simulink model "wlan_phy_rx_pmd"  (Simulink 7.8)
settings: Solver=VariableStepDiscrete  SolverMode=SingleTasking  StartTime=0.0  StopTime=rx_sim.sim_time  RelTol=1e-3
PostLoadFcn:
  | wlan_phy_rx_init
InitFcn:
  | wlan_phy_rx_init
StartFcn:
  | wlan_phy_rx_init
values from neighbouring files (symbols this model uses):
  barker_seq20 = 0.95 * circshift(barker_seq20, [0 4]) ./ max(abs(barker_seq20))    [wlan_phy_rx_init.m]
  bit_scrambler_lfsr_bytes = sum(bit_scrambler_lfsr_bytes .* repmat(2.^[0:7], 127, 1),2)    [wlan_phy_rx_init.m]
  longCorr_coef_i = [3*real(longCorr_coef)]    [wlan_phy_rx_init.m]
  longCorr_coef_q = [3*imag(longCorr_coef)]    [wlan_phy_rx_init.m]
  MAX_CP_LEN = 32    [wlan_phy_rx_init.m]
  MAX_NCBPS = NCBPS_11n_64QAM    (= 312)    [wlan_phy_rx_init.m]
  MAX_NUM_BYTES = 2^16-1    (= 65535)    [wlan_phy_rx_init.m]
  MAX_NUM_SC = 64    [wlan_phy_rx_init.m]
  MAX_NUM_SYMS = 600    [wlan_phy_rx_init.m]
  NDBPS_11a_BPSK12 = 24    [wlan_phy_rx_init.m]
  preFFT_sampBuff_numSamps = 4*MAX_NUM_SC    (= 256)    [wlan_phy_rx_init.m]
  rx_sim = struct()    [wlan_phy_rx_init.m]
  scr_ind_rev = zeros(1,128)    [wlan_phy_rx_init.m]
undefined symbols (not defined in the file or in its folder): gcbh, mcs_rom_11ag, mcs_rom_11n, wlan_rx_deinterleave_rom_gen, xlBlockLoadCallback, xlGetNormalizedPeriod, xlipgetpartsetting
libraries: xbsIndex_r4 x4943, xbsEDKLib_r4 x1
(part 25 of 28)

== system wlan_phy_rx_pmd/Sync & Antenna Sel/LTS Correlation/Correlator/C3/C3/MAC ==
  a : Inport
  coeff : Inport  Port=2
  ld : Inport  Port=3
  rst : Inport  Port=4
  AddSub : xbsIndex_r4/AddSub  mode=Addition or Subtraction  use_carryin=off  use_carryout=off  en=off  latency=0  precision=User Defined  arith_type="Signed  (2's comp)"  n_bits=long_cor_acc_n_bits  bin_pt=0  quantization=Truncate  overflow=Flag as error  dbl_ovrd=off  use_behavioral_HDL=on  hw_selection=Fabric  pipelined=off  use_rpm=off  block_type=addsub
      settings: xl_area=[2 0 0 4 0 0 0]  block_version=VER_STRING_GOES_HERE
  Gateway Out{1..3,6..8} (6 alike) : xbsIndex_r4/Gateway Out  inherit_from_input=off  hdl_port=off  timing_constraint=None  locs_specified=off  LOCs={}  UseAsDAC=off  DACChannel='1'  block_type=gatewayout
      settings: infoedit="Gateway out block.  Converts Xilinx fixed point inputs into ouputs of type Simulink integer, double, or fixed point.<P><P>Hardware notes:  In hardware these blocks become top level output ports or are discarded, depending on how they are configured."  xl_area=[0 0 0 0 0 0 0]  block_version=VER_STRING_GOES_HERE
  Register1 : xbsIndex_r4/Register  init=0  rst=on  en=off  dbl_ovrd=off  block_type=register
      settings: xl_area=[2 4 0 0 0 0 0]  block_version=VER_STRING_GOES_HERE
  Register2 : xbsIndex_r4/Register  init=0  rst=off  en=on  dbl_ovrd=off  block_type=register
      settings: xl_area=[2 4 0 0 0 0 0]  block_version=VER_STRING_GOES_HERE
  bc2 : xbsIndex_r4/Convert  gui_display_data_type=Fixed-point  arith_type=Boolean  n_bits=1  bin_pt=0  float_type=Single  exp_bits=8  fraction_bits=24  quantization=Truncate  overflow=Wrap  en=off  latency=0  dbl_ovrd=off  pipeline=off  block_type=convert
      settings: xl_area=[0 0 0 0 0 0 0]  block_version=VER_STRING_GOES_HERE
  bc3 : xbsIndex_r4/Convert  gui_display_data_type=Fixed-point  arith_type=Boolean  n_bits=1  bin_pt=0  float_type=Single  exp_bits=8  fraction_bits=24  quantization=Truncate  overflow=Wrap  en=off  latency=0  dbl_ovrd=off  pipeline=off  block_type=convert
      settings: xl_area=[0 0 0 0 0 0 0]  block_version=VER_STRING_GOES_HERE
  yn : Outport
  accrm : Outport  Port=2
  wires:
  rst/1 -> Gateway Out1/1, Register1/2
  Gateway Out3/1 -> ?
  Gateway Out2/1 -> ?
  Gateway Out1/1 -> ?
  Gateway Out6/1 -> ?
  Gateway Out7/1 -> ?
  Gateway Out8/1 -> ?
  a/1 -> Gateway Out7/1, bc2/1
  AddSub/1 -> Gateway Out8/1, accrm/1, Register1/1, Register2/1
  Register2/1 -> Gateway Out3/1, yn/1
  Register1/1 -> AddSub/1
  ld/1 -> Gateway Out2/1, bc3/1
  bc3/1 -> Register2/2
  bc2/1 -> AddSub/3
  coeff/1 -> Gateway Out6/1, AddSub/2

== system wlan_phy_rx_pmd/Sync & Antenna Sel/LTS Correlation/Correlator/C3/C4/MAC ==
  a : Inport
  coeff : Inport  Port=2
  ld : Inport  Port=3
  rst : Inport  Port=4
  AddSub : xbsIndex_r4/AddSub  mode=Addition or Subtraction  use_carryin=off  use_carryout=off  en=off  latency=0  precision=User Defined  arith_type="Signed  (2's comp)"  n_bits=long_cor_acc_n_bits  bin_pt=0  quantization=Truncate  overflow=Flag as error  dbl_ovrd=off  use_behavioral_HDL=on  hw_selection=Fabric  pipelined=off  use_rpm=off  block_type=addsub
      settings: xl_area=[2 0 0 4 0 0 0]  block_version=VER_STRING_GOES_HERE
  Gateway Out{1..3,6..8} (6 alike) : xbsIndex_r4/Gateway Out  inherit_from_input=off  hdl_port=off  timing_constraint=None  locs_specified=off  LOCs={}  UseAsDAC=off  DACChannel='1'  block_type=gatewayout
      settings: infoedit="Gateway out block.  Converts Xilinx fixed point inputs into ouputs of type Simulink integer, double, or fixed point.<P><P>Hardware notes:  In hardware these blocks become top level output ports or are discarded, depending on how they are configured."  xl_area=[0 0 0 0 0 0 0]  block_version=VER_STRING_GOES_HERE
  Register1 : xbsIndex_r4/Register  init=0  rst=on  en=off  dbl_ovrd=off  block_type=register
      settings: xl_area=[2 4 0 0 0 0 0]  block_version=VER_STRING_GOES_HERE
  Register2 : xbsIndex_r4/Register  init=0  rst=off  en=on  dbl_ovrd=off  block_type=register
      settings: xl_area=[2 4 0 0 0 0 0]  block_version=VER_STRING_GOES_HERE
  bc2 : xbsIndex_r4/Convert  gui_display_data_type=Fixed-point  arith_type=Boolean  n_bits=1  bin_pt=0  float_type=Single  exp_bits=8  fraction_bits=24  quantization=Truncate  overflow=Wrap  en=off  latency=0  dbl_ovrd=off  pipeline=off  block_type=convert
      settings: xl_area=[0 0 0 0 0 0 0]  block_version=VER_STRING_GOES_HERE
  bc3 : xbsIndex_r4/Convert  gui_display_data_type=Fixed-point  arith_type=Boolean  n_bits=1  bin_pt=0  float_type=Single  exp_bits=8  fraction_bits=24  quantization=Truncate  overflow=Wrap  en=off  latency=0  dbl_ovrd=off  pipeline=off  block_type=convert
      settings: xl_area=[0 0 0 0 0 0 0]  block_version=VER_STRING_GOES_HERE
  yn : Outport
  accrm : Outport  Port=2
  wires:
  coeff/1 -> AddSub/2, Gateway Out6/1
  bc2/1 -> AddSub/3
  bc3/1 -> Register2/2
  ld/1 -> bc3/1, Gateway Out2/1
  Register1/1 -> AddSub/1
  Register2/1 -> yn/1, Gateway Out3/1
  AddSub/1 -> Register2/1, Register1/1, accrm/1, Gateway Out8/1
  a/1 -> bc2/1, Gateway Out7/1
  Gateway Out8/1 -> ?
  Gateway Out7/1 -> ?
  Gateway Out6/1 -> ?
  Gateway Out1/1 -> ?
  Gateway Out2/1 -> ?
  Gateway Out3/1 -> ?
  rst/1 -> Register1/2, Gateway Out1/1

== system wlan_phy_rx_pmd/Sync & Antenna Sel/LTS Correlation/Correlator/C3/C5/MAC ==
  a : Inport
  coeff : Inport  Port=2
  ld : Inport  Port=3
  rst : Inport  Port=4
  AddSub : xbsIndex_r4/AddSub  mode=Addition or Subtraction  use_carryin=off  use_carryout=off  en=off  latency=0  precision=User Defined  arith_type="Signed  (2's comp)"  n_bits=long_cor_acc_n_bits  bin_pt=0  quantization=Truncate  overflow=Flag as error  dbl_ovrd=off  use_behavioral_HDL=on  hw_selection=Fabric  pipelined=off  use_rpm=off  block_type=addsub
      settings: xl_area=[2 0 0 4 0 0 0]  block_version=VER_STRING_GOES_HERE
  Gateway Out{1..3,6..8} (6 alike) : xbsIndex_r4/Gateway Out  inherit_from_input=off  hdl_port=off  timing_constraint=None  locs_specified=off  LOCs={}  UseAsDAC=off  DACChannel='1'  block_type=gatewayout
      settings: infoedit="Gateway out block.  Converts Xilinx fixed point inputs into ouputs of type Simulink integer, double, or fixed point.<P><P>Hardware notes:  In hardware these blocks become top level output ports or are discarded, depending on how they are configured."  xl_area=[0 0 0 0 0 0 0]  block_version=VER_STRING_GOES_HERE
  Register1 : xbsIndex_r4/Register  init=0  rst=on  en=off  dbl_ovrd=off  block_type=register
      settings: xl_area=[2 4 0 0 0 0 0]  block_version=VER_STRING_GOES_HERE
  Register2 : xbsIndex_r4/Register  init=0  rst=off  en=on  dbl_ovrd=off  block_type=register
      settings: xl_area=[2 4 0 0 0 0 0]  block_version=VER_STRING_GOES_HERE
  bc2 : xbsIndex_r4/Convert  gui_display_data_type=Fixed-point  arith_type=Boolean  n_bits=1  bin_pt=0  float_type=Single  exp_bits=8  fraction_bits=24  quantization=Truncate  overflow=Wrap  en=off  latency=0  dbl_ovrd=off  pipeline=off  block_type=convert
      settings: xl_area=[0 0 0 0 0 0 0]  block_version=VER_STRING_GOES_HERE
  bc3 : xbsIndex_r4/Convert  gui_display_data_type=Fixed-point  arith_type=Boolean  n_bits=1  bin_pt=0  float_type=Single  exp_bits=8  fraction_bits=24  quantization=Truncate  overflow=Wrap  en=off  latency=0  dbl_ovrd=off  pipeline=off  block_type=convert
      settings: xl_area=[0 0 0 0 0 0 0]  block_version=VER_STRING_GOES_HERE
  yn : Outport
  accrm : Outport  Port=2
  wires:
  rst/1 -> Gateway Out1/1, Register1/2
  Gateway Out3/1 -> ?
  Gateway Out2/1 -> ?
  Gateway Out1/1 -> ?
  Gateway Out6/1 -> ?
  Gateway Out7/1 -> ?
  Gateway Out8/1 -> ?
  a/1 -> Gateway Out7/1, bc2/1
  AddSub/1 -> Gateway Out8/1, accrm/1, Register1/1, Register2/1
  Register2/1 -> Gateway Out3/1, yn/1
  Register1/1 -> AddSub/1
  ld/1 -> Gateway Out2/1, bc3/1
  bc3/1 -> Register2/2
  bc2/1 -> AddSub/3
  coeff/1 -> Gateway Out6/1, AddSub/2

== system wlan_phy_rx_pmd/Sync & Antenna Sel/LTS Correlation/Correlator/C3/C6/MAC ==
  a : Inport
  coeff : Inport  Port=2
  ld : Inport  Port=3
  rst : Inport  Port=4
  AddSub : xbsIndex_r4/AddSub  mode=Addition or Subtraction  use_carryin=off  use_carryout=off  en=off  latency=0  precision=User Defined  arith_type="Signed  (2's comp)"  n_bits=long_cor_acc_n_bits  bin_pt=0  quantization=Truncate  overflow=Flag as error  dbl_ovrd=off  use_behavioral_HDL=on  hw_selection=Fabric  pipelined=off  use_rpm=off  block_type=addsub
      settings: xl_area=[2 0 0 4 0 0 0]  block_version=VER_STRING_GOES_HERE
  Gateway Out{1..3,6..8} (6 alike) : xbsIndex_r4/Gateway Out  inherit_from_input=off  hdl_port=off  timing_constraint=None  locs_specified=off  LOCs={}  UseAsDAC=off  DACChannel='1'  block_type=gatewayout
      settings: infoedit="Gateway out block.  Converts Xilinx fixed point inputs into ouputs of type Simulink integer, double, or fixed point.<P><P>Hardware notes:  In hardware these blocks become top level output ports or are discarded, depending on how they are configured."  xl_area=[0 0 0 0 0 0 0]  block_version=VER_STRING_GOES_HERE
  Register1 : xbsIndex_r4/Register  init=0  rst=on  en=off  dbl_ovrd=off  block_type=register
      settings: xl_area=[2 4 0 0 0 0 0]  block_version=VER_STRING_GOES_HERE
  Register2 : xbsIndex_r4/Register  init=0  rst=off  en=on  dbl_ovrd=off  block_type=register
      settings: xl_area=[2 4 0 0 0 0 0]  block_version=VER_STRING_GOES_HERE
  bc2 : xbsIndex_r4/Convert  gui_display_data_type=Fixed-point  arith_type=Boolean  n_bits=1  bin_pt=0  float_type=Single  exp_bits=8  fraction_bits=24  quantization=Truncate  overflow=Wrap  en=off  latency=0  dbl_ovrd=off  pipeline=off  block_type=convert
      settings: xl_area=[0 0 0 0 0 0 0]  block_version=VER_STRING_GOES_HERE
  bc3 : xbsIndex_r4/Convert  gui_display_data_type=Fixed-point  arith_type=Boolean  n_bits=1  bin_pt=0  float_type=Single  exp_bits=8  fraction_bits=24  quantization=Truncate  overflow=Wrap  en=off  latency=0  dbl_ovrd=off  pipeline=off  block_type=convert
      settings: xl_area=[0 0 0 0 0 0 0]  block_version=VER_STRING_GOES_HERE
  yn : Outport
  accrm : Outport  Port=2
  wires:
  coeff/1 -> AddSub/2, Gateway Out6/1
  bc2/1 -> AddSub/3
  bc3/1 -> Register2/2
  ld/1 -> bc3/1, Gateway Out2/1
  Register1/1 -> AddSub/1
  Register2/1 -> yn/1, Gateway Out3/1
  AddSub/1 -> Register2/1, Register1/1, accrm/1, Gateway Out8/1
  a/1 -> bc2/1, Gateway Out7/1
  Gateway Out8/1 -> ?
  Gateway Out7/1 -> ?
  Gateway Out6/1 -> ?
  Gateway Out1/1 -> ?
  Gateway Out2/1 -> ?
  Gateway Out3/1 -> ?
  rst/1 -> Register1/2, Gateway Out1/1

== system wlan_phy_rx_pmd/Sync & Antenna Sel/LTS Correlation/Correlator/C3/C7/MAC ==
  a : Inport
  coeff : Inport  Port=2
  ld : Inport  Port=3
  rst : Inport  Port=4
  AddSub : xbsIndex_r4/AddSub  mode=Addition or Subtraction  use_carryin=off  use_carryout=off  en=off  latency=0  precision=User Defined  arith_type="Signed  (2's comp)"  n_bits=long_cor_acc_n_bits  bin_pt=0  quantization=Truncate  overflow=Flag as error  dbl_ovrd=off  use_behavioral_HDL=on  hw_selection=Fabric  pipelined=off  use_rpm=off  block_type=addsub
      settings: xl_area=[2 0 0 4 0 0 0]  block_version=VER_STRING_GOES_HERE
  Gateway Out{1..3,6..8} (6 alike) : xbsIndex_r4/Gateway Out  inherit_from_input=off  hdl_port=off  timing_constraint=None  locs_specified=off  LOCs={}  UseAsDAC=off  DACChannel='1'  block_type=gatewayout
      settings: infoedit="Gateway out block.  Converts Xilinx fixed point inputs into ouputs of type Simulink integer, double, or fixed point.<P><P>Hardware notes:  In hardware these blocks become top level output ports or are discarded, depending on how they are configured."  xl_area=[0 0 0 0 0 0 0]  block_version=VER_STRING_GOES_HERE
  Register1 : xbsIndex_r4/Register  init=0  rst=on  en=off  dbl_ovrd=off  block_type=register
      settings: xl_area=[2 4 0 0 0 0 0]  block_version=VER_STRING_GOES_HERE
  Register2 : xbsIndex_r4/Register  init=0  rst=off  en=on  dbl_ovrd=off  block_type=register
      settings: xl_area=[2 4 0 0 0 0 0]  block_version=VER_STRING_GOES_HERE
  bc2 : xbsIndex_r4/Convert  gui_display_data_type=Fixed-point  arith_type=Boolean  n_bits=1  bin_pt=0  float_type=Single  exp_bits=8  fraction_bits=24  quantization=Truncate  overflow=Wrap  en=off  latency=0  dbl_ovrd=off  pipeline=off  block_type=convert
      settings: xl_area=[0 0 0 0 0 0 0]  block_version=VER_STRING_GOES_HERE
  bc3 : xbsIndex_r4/Convert  gui_display_data_type=Fixed-point  arith_type=Boolean  n_bits=1  bin_pt=0  float_type=Single  exp_bits=8  fraction_bits=24  quantization=Truncate  overflow=Wrap  en=off  latency=0  dbl_ovrd=off  pipeline=off  block_type=convert
      settings: xl_area=[0 0 0 0 0 0 0]  block_version=VER_STRING_GOES_HERE
  yn : Outport
  accrm : Outport  Port=2
  wires:
  rst/1 -> Gateway Out1/1, Register1/2
  Gateway Out3/1 -> ?
  Gateway Out2/1 -> ?
  Gateway Out1/1 -> ?
  Gateway Out6/1 -> ?
  Gateway Out7/1 -> ?
  Gateway Out8/1 -> ?
  a/1 -> Gateway Out7/1, bc2/1
  AddSub/1 -> Gateway Out8/1, accrm/1, Register1/1, Register2/1
  Register2/1 -> Gateway Out3/1, yn/1
  Register1/1 -> AddSub/1
  ld/1 -> Gateway Out2/1, bc3/1
  bc3/1 -> Register2/2
  bc2/1 -> AddSub/3
  coeff/1 -> Gateway Out6/1, AddSub/2

== system wlan_phy_rx_pmd/Sync & Antenna Sel/LTS Correlation/Correlator/C3/C8/MAC ==
  a : Inport
  coeff : Inport  Port=2
  ld : Inport  Port=3
  rst : Inport  Port=4
  AddSub : xbsIndex_r4/AddSub  mode=Addition or Subtraction  use_carryin=off  use_carryout=off  en=off  latency=0  precision=User Defined  arith_type="Signed  (2's comp)"  n_bits=long_cor_acc_n_bits  bin_pt=0  quantization=Truncate  overflow=Flag as error  dbl_ovrd=off  use_behavioral_HDL=on  hw_selection=Fabric  pipelined=off  use_rpm=off  block_type=addsub
      settings: xl_area=[2 0 0 4 0 0 0]  block_version=VER_STRING_GOES_HERE
  Gateway Out{1..3,6..8} (6 alike) : xbsIndex_r4/Gateway Out  inherit_from_input=off  hdl_port=off  timing_constraint=None  locs_specified=off  LOCs={}  UseAsDAC=off  DACChannel='1'  block_type=gatewayout
      settings: infoedit="Gateway out block.  Converts Xilinx fixed point inputs into ouputs of type Simulink integer, double, or fixed point.<P><P>Hardware notes:  In hardware these blocks become top level output ports or are discarded, depending on how they are configured."  xl_area=[0 0 0 0 0 0 0]  block_version=VER_STRING_GOES_HERE
  Register1 : xbsIndex_r4/Register  init=0  rst=on  en=off  dbl_ovrd=off  block_type=register
      settings: xl_area=[2 4 0 0 0 0 0]  block_version=VER_STRING_GOES_HERE
  Register2 : xbsIndex_r4/Register  init=0  rst=off  en=on  dbl_ovrd=off  block_type=register
      settings: xl_area=[2 4 0 0 0 0 0]  block_version=VER_STRING_GOES_HERE
  bc2 : xbsIndex_r4/Convert  gui_display_data_type=Fixed-point  arith_type=Boolean  n_bits=1  bin_pt=0  float_type=Single  exp_bits=8  fraction_bits=24  quantization=Truncate  overflow=Wrap  en=off  latency=0  dbl_ovrd=off  pipeline=off  block_type=convert
      settings: xl_area=[0 0 0 0 0 0 0]  block_version=VER_STRING_GOES_HERE
  bc3 : xbsIndex_r4/Convert  gui_display_data_type=Fixed-point  arith_type=Boolean  n_bits=1  bin_pt=0  float_type=Single  exp_bits=8  fraction_bits=24  quantization=Truncate  overflow=Wrap  en=off  latency=0  dbl_ovrd=off  pipeline=off  block_type=convert
      settings: xl_area=[0 0 0 0 0 0 0]  block_version=VER_STRING_GOES_HERE
  yn : Outport
  accrm : Outport  Port=2
  wires:
  coeff/1 -> AddSub/2, Gateway Out6/1
  bc2/1 -> AddSub/3
  bc3/1 -> Register2/2
  ld/1 -> bc3/1, Gateway Out2/1
  Register1/1 -> AddSub/1
  Register2/1 -> yn/1, Gateway Out3/1
  AddSub/1 -> Register2/1, Register1/1, accrm/1, Gateway Out8/1
  a/1 -> bc2/1, Gateway Out7/1
  Gateway Out8/1 -> ?
  Gateway Out7/1 -> ?
  Gateway Out6/1 -> ?
  Gateway Out1/1 -> ?
  Gateway Out2/1 -> ?
  Gateway Out3/1 -> ?
  rst/1 -> Register1/2, Gateway Out1/1

== system wlan_phy_rx_pmd/Sync & Antenna Sel/LTS Correlation/Correlator/C4/C1/MAC ==
  a : Inport
  coeff : Inport  Port=2
  ld : Inport  Port=3
  rst : Inport  Port=4
  AddSub : xbsIndex_r4/AddSub  mode=Addition or Subtraction  use_carryin=off  use_carryout=off  en=off  latency=0  precision=User Defined  arith_type="Signed  (2's comp)"  n_bits=long_cor_acc_n_bits  bin_pt=0  quantization=Truncate  overflow=Flag as error  dbl_ovrd=off  use_behavioral_HDL=on  hw_selection=Fabric  pipelined=off  use_rpm=off  block_type=addsub
      settings: xl_area=[2 0 0 4 0 0 0]  block_version=VER_STRING_GOES_HERE
  Gateway Out{1..3,6..8} (6 alike) : xbsIndex_r4/Gateway Out  inherit_from_input=off  hdl_port=off  timing_constraint=None  locs_specified=off  LOCs={}  UseAsDAC=off  DACChannel='1'  block_type=gatewayout
      settings: infoedit="Gateway out block.  Converts Xilinx fixed point inputs into ouputs of type Simulink integer, double, or fixed point.<P><P>Hardware notes:  In hardware these blocks become top level output ports or are discarded, depending on how they are configured."  xl_area=[0 0 0 0 0 0 0]  block_version=VER_STRING_GOES_HERE
  Register1 : xbsIndex_r4/Register  init=0  rst=on  en=off  dbl_ovrd=off  block_type=register
      settings: xl_area=[2 4 0 0 0 0 0]  block_version=VER_STRING_GOES_HERE
  Register2 : xbsIndex_r4/Register  init=0  rst=off  en=on  dbl_ovrd=off  block_type=register
      settings: xl_area=[2 4 0 0 0 0 0]  block_version=VER_STRING_GOES_HERE
  bc2 : xbsIndex_r4/Convert  gui_display_data_type=Fixed-point  arith_type=Boolean  n_bits=1  bin_pt=0  float_type=Single  exp_bits=8  fraction_bits=24  quantization=Truncate  overflow=Wrap  en=off  latency=0  dbl_ovrd=off  pipeline=off  block_type=convert
      settings: xl_area=[0 0 0 0 0 0 0]  block_version=VER_STRING_GOES_HERE
  bc3 : xbsIndex_r4/Convert  gui_display_data_type=Fixed-point  arith_type=Boolean  n_bits=1  bin_pt=0  float_type=Single  exp_bits=8  fraction_bits=24  quantization=Truncate  overflow=Wrap  en=off  latency=0  dbl_ovrd=off  pipeline=off  block_type=convert
      settings: xl_area=[0 0 0 0 0 0 0]  block_version=VER_STRING_GOES_HERE
  yn : Outport
  accrm : Outport  Port=2
  wires:
  coeff/1 -> AddSub/2, Gateway Out6/1
  bc2/1 -> AddSub/3
  bc3/1 -> Register2/2
  ld/1 -> bc3/1, Gateway Out2/1
  Register1/1 -> AddSub/1
  Register2/1 -> yn/1, Gateway Out3/1
  AddSub/1 -> Register2/1, Register1/1, accrm/1, Gateway Out8/1
  a/1 -> bc2/1, Gateway Out7/1
  Gateway Out8/1 -> ?
  Gateway Out7/1 -> ?
  Gateway Out6/1 -> ?
  Gateway Out1/1 -> ?
  Gateway Out2/1 -> ?
  Gateway Out3/1 -> ?
  rst/1 -> Register1/2, Gateway Out1/1

== system wlan_phy_rx_pmd/Sync & Antenna Sel/LTS Correlation/Correlator/C4/C2/MAC ==
  a : Inport
  coeff : Inport  Port=2
  ld : Inport  Port=3
  rst : Inport  Port=4
  AddSub : xbsIndex_r4/AddSub  mode=Addition or Subtraction  use_carryin=off  use_carryout=off  en=off  latency=0  precision=User Defined  arith_type="Signed  (2's comp)"  n_bits=long_cor_acc_n_bits  bin_pt=0  quantization=Truncate  overflow=Flag as error  dbl_ovrd=off  use_behavioral_HDL=on  hw_selection=Fabric  pipelined=off  use_rpm=off  block_type=addsub
      settings: xl_area=[2 0 0 4 0 0 0]  block_version=VER_STRING_GOES_HERE
  Gateway Out{1..3,6..8} (6 alike) : xbsIndex_r4/Gateway Out  inherit_from_input=off  hdl_port=off  timing_constraint=None  locs_specified=off  LOCs={}  UseAsDAC=off  DACChannel='1'  block_type=gatewayout
      settings: infoedit="Gateway out block.  Converts Xilinx fixed point inputs into ouputs of type Simulink integer, double, or fixed point.<P><P>Hardware notes:  In hardware these blocks become top level output ports or are discarded, depending on how they are configured."  xl_area=[0 0 0 0 0 0 0]  block_version=VER_STRING_GOES_HERE
  Register1 : xbsIndex_r4/Register  init=0  rst=on  en=off  dbl_ovrd=off  block_type=register
      settings: xl_area=[2 4 0 0 0 0 0]  block_version=VER_STRING_GOES_HERE
  Register2 : xbsIndex_r4/Register  init=0  rst=off  en=on  dbl_ovrd=off  block_type=register
      settings: xl_area=[2 4 0 0 0 0 0]  block_version=VER_STRING_GOES_HERE
  bc2 : xbsIndex_r4/Convert  gui_display_data_type=Fixed-point  arith_type=Boolean  n_bits=1  bin_pt=0  float_type=Single  exp_bits=8  fraction_bits=24  quantization=Truncate  overflow=Wrap  en=off  latency=0  dbl_ovrd=off  pipeline=off  block_type=convert
      settings: xl_area=[0 0 0 0 0 0 0]  block_version=VER_STRING_GOES_HERE
  bc3 : xbsIndex_r4/Convert  gui_display_data_type=Fixed-point  arith_type=Boolean  n_bits=1  bin_pt=0  float_type=Single  exp_bits=8  fraction_bits=24  quantization=Truncate  overflow=Wrap  en=off  latency=0  dbl_ovrd=off  pipeline=off  block_type=convert
      settings: xl_area=[0 0 0 0 0 0 0]  block_version=VER_STRING_GOES_HERE
  yn : Outport
  accrm : Outport  Port=2
  wires:
  rst/1 -> Gateway Out1/1, Register1/2
  Gateway Out3/1 -> ?
  Gateway Out2/1 -> ?
  Gateway Out1/1 -> ?
  Gateway Out6/1 -> ?
  Gateway Out7/1 -> ?
  Gateway Out8/1 -> ?
  a/1 -> Gateway Out7/1, bc2/1
  AddSub/1 -> Gateway Out8/1, accrm/1, Register1/1, Register2/1
  Register2/1 -> Gateway Out3/1, yn/1
  Register1/1 -> AddSub/1
  ld/1 -> Gateway Out2/1, bc3/1
  bc3/1 -> Register2/2
  bc2/1 -> AddSub/3
  coeff/1 -> Gateway Out6/1, AddSub/2

== system wlan_phy_rx_pmd/Sync & Antenna Sel/LTS Correlation/Correlator/C4/C3/MAC ==
  a : Inport
  coeff : Inport  Port=2
  ld : Inport  Port=3
  rst : Inport  Port=4
  AddSub : xbsIndex_r4/AddSub  mode=Addition or Subtraction  use_carryin=off  use_carryout=off  en=off  latency=0  precision=User Defined  arith_type="Signed  (2's comp)"  n_bits=long_cor_acc_n_bits  bin_pt=0  quantization=Truncate  overflow=Flag as error  dbl_ovrd=off  use_behavioral_HDL=on  hw_selection=Fabric  pipelined=off  use_rpm=off  block_type=addsub
      settings: xl_area=[2 0 0 4 0 0 0]  block_version=VER_STRING_GOES_HERE
  Gateway Out{1..3,6..8} (6 alike) : xbsIndex_r4/Gateway Out  inherit_from_input=off  hdl_port=off  timing_constraint=None  locs_specified=off  LOCs={}  UseAsDAC=off  DACChannel='1'  block_type=gatewayout
      settings: infoedit="Gateway out block.  Converts Xilinx fixed point inputs into ouputs of type Simulink integer, double, or fixed point.<P><P>Hardware notes:  In hardware these blocks become top level output ports or are discarded, depending on how they are configured."  xl_area=[0 0 0 0 0 0 0]  block_version=VER_STRING_GOES_HERE
  Register1 : xbsIndex_r4/Register  init=0  rst=on  en=off  dbl_ovrd=off  block_type=register
      settings: xl_area=[2 4 0 0 0 0 0]  block_version=VER_STRING_GOES_HERE
  Register2 : xbsIndex_r4/Register  init=0  rst=off  en=on  dbl_ovrd=off  block_type=register
      settings: xl_area=[2 4 0 0 0 0 0]  block_version=VER_STRING_GOES_HERE
  bc2 : xbsIndex_r4/Convert  gui_display_data_type=Fixed-point  arith_type=Boolean  n_bits=1  bin_pt=0  float_type=Single  exp_bits=8  fraction_bits=24  quantization=Truncate  overflow=Wrap  en=off  latency=0  dbl_ovrd=off  pipeline=off  block_type=convert
      settings: xl_area=[0 0 0 0 0 0 0]  block_version=VER_STRING_GOES_HERE
  bc3 : xbsIndex_r4/Convert  gui_display_data_type=Fixed-point  arith_type=Boolean  n_bits=1  bin_pt=0  float_type=Single  exp_bits=8  fraction_bits=24  quantization=Truncate  overflow=Wrap  en=off  latency=0  dbl_ovrd=off  pipeline=off  block_type=convert
      settings: xl_area=[0 0 0 0 0 0 0]  block_version=VER_STRING_GOES_HERE
  yn : Outport
  accrm : Outport  Port=2
  wires:
  coeff/1 -> AddSub/2, Gateway Out6/1
  bc2/1 -> AddSub/3
  bc3/1 -> Register2/2
  ld/1 -> bc3/1, Gateway Out2/1
  Register1/1 -> AddSub/1
  Register2/1 -> yn/1, Gateway Out3/1
  AddSub/1 -> Register2/1, Register1/1, accrm/1, Gateway Out8/1
  a/1 -> bc2/1, Gateway Out7/1
  Gateway Out8/1 -> ?
  Gateway Out7/1 -> ?
  Gateway Out6/1 -> ?
  Gateway Out1/1 -> ?
  Gateway Out2/1 -> ?
  Gateway Out3/1 -> ?
  rst/1 -> Register1/2, Gateway Out1/1

== system wlan_phy_rx_pmd/Sync & Antenna Sel/LTS Correlation/Correlator/C4/C4/MAC ==
  a : Inport
  coeff : Inport  Port=2
  ld : Inport  Port=3
  rst : Inport  Port=4
  AddSub : xbsIndex_r4/AddSub  mode=Addition or Subtraction  use_carryin=off  use_carryout=off  en=off  latency=0  precision=User Defined  arith_type="Signed  (2's comp)"  n_bits=long_cor_acc_n_bits  bin_pt=0  quantization=Truncate  overflow=Flag as error  dbl_ovrd=off  use_behavioral_HDL=on  hw_selection=Fabric  pipelined=off  use_rpm=off  block_type=addsub
      settings: xl_area=[2 0 0 4 0 0 0]  block_version=VER_STRING_GOES_HERE
  Gateway Out{1..3,6..8} (6 alike) : xbsIndex_r4/Gateway Out  inherit_from_input=off  hdl_port=off  timing_constraint=None  locs_specified=off  LOCs={}  UseAsDAC=off  DACChannel='1'  block_type=gatewayout
      settings: infoedit="Gateway out block.  Converts Xilinx fixed point inputs into ouputs of type Simulink integer, double, or fixed point.<P><P>Hardware notes:  In hardware these blocks become top level output ports or are discarded, depending on how they are configured."  xl_area=[0 0 0 0 0 0 0]  block_version=VER_STRING_GOES_HERE
  Register1 : xbsIndex_r4/Register  init=0  rst=on  en=off  dbl_ovrd=off  block_type=register
      settings: xl_area=[2 4 0 0 0 0 0]  block_version=VER_STRING_GOES_HERE
  Register2 : xbsIndex_r4/Register  init=0  rst=off  en=on  dbl_ovrd=off  block_type=register
      settings: xl_area=[2 4 0 0 0 0 0]  block_version=VER_STRING_GOES_HERE
  bc2 : xbsIndex_r4/Convert  gui_display_data_type=Fixed-point  arith_type=Boolean  n_bits=1  bin_pt=0  float_type=Single  exp_bits=8  fraction_bits=24  quantization=Truncate  overflow=Wrap  en=off  latency=0  dbl_ovrd=off  pipeline=off  block_type=convert
      settings: xl_area=[0 0 0 0 0 0 0]  block_version=VER_STRING_GOES_HERE
  bc3 : xbsIndex_r4/Convert  gui_display_data_type=Fixed-point  arith_type=Boolean  n_bits=1  bin_pt=0  float_type=Single  exp_bits=8  fraction_bits=24  quantization=Truncate  overflow=Wrap  en=off  latency=0  dbl_ovrd=off  pipeline=off  block_type=convert
      settings: xl_area=[0 0 0 0 0 0 0]  block_version=VER_STRING_GOES_HERE
  yn : Outport
  accrm : Outport  Port=2
  wires:
  rst/1 -> Gateway Out1/1, Register1/2
  Gateway Out3/1 -> ?
  Gateway Out2/1 -> ?
  Gateway Out1/1 -> ?
  Gateway Out6/1 -> ?
  Gateway Out7/1 -> ?
  Gateway Out8/1 -> ?
  a/1 -> Gateway Out7/1, bc2/1
  AddSub/1 -> Gateway Out8/1, accrm/1, Register1/1, Register2/1
  Register2/1 -> Gateway Out3/1, yn/1
  Register1/1 -> AddSub/1
  ld/1 -> Gateway Out2/1, bc3/1
  bc3/1 -> Register2/2
  bc2/1 -> AddSub/3
  coeff/1 -> Gateway Out6/1, AddSub/2

== system wlan_phy_rx_pmd/Sync & Antenna Sel/LTS Correlation/Correlator/C4/C5/MAC ==
  a : Inport
  coeff : Inport  Port=2
  ld : Inport  Port=3
  rst : Inport  Port=4
  AddSub : xbsIndex_r4/AddSub  mode=Addition or Subtraction  use_carryin=off  use_carryout=off  en=off  latency=0  precision=User Defined  arith_type="Signed  (2's comp)"  n_bits=long_cor_acc_n_bits  bin_pt=0  quantization=Truncate  overflow=Flag as error  dbl_ovrd=off  use_behavioral_HDL=on  hw_selection=Fabric  pipelined=off  use_rpm=off  block_type=addsub
      settings: xl_area=[2 0 0 4 0 0 0]  block_version=VER_STRING_GOES_HERE
  Gateway Out{1..3,6..8} (6 alike) : xbsIndex_r4/Gateway Out  inherit_from_input=off  hdl_port=off  timing_constraint=None  locs_specified=off  LOCs={}  UseAsDAC=off  DACChannel='1'  block_type=gatewayout
      settings: infoedit="Gateway out block.  Converts Xilinx fixed point inputs into ouputs of type Simulink integer, double, or fixed point.<P><P>Hardware notes:  In hardware these blocks become top level output ports or are discarded, depending on how they are configured."  xl_area=[0 0 0 0 0 0 0]  block_version=VER_STRING_GOES_HERE
  Register1 : xbsIndex_r4/Register  init=0  rst=on  en=off  dbl_ovrd=off  block_type=register
      settings: xl_area=[2 4 0 0 0 0 0]  block_version=VER_STRING_GOES_HERE
  Register2 : xbsIndex_r4/Register  init=0  rst=off  en=on  dbl_ovrd=off  block_type=register
      settings: xl_area=[2 4 0 0 0 0 0]  block_version=VER_STRING_GOES_HERE
  bc2 : xbsIndex_r4/Convert  gui_display_data_type=Fixed-point  arith_type=Boolean  n_bits=1  bin_pt=0  float_type=Single  exp_bits=8  fraction_bits=24  quantization=Truncate  overflow=Wrap  en=off  latency=0  dbl_ovrd=off  pipeline=off  block_type=convert
      settings: xl_area=[0 0 0 0 0 0 0]  block_version=VER_STRING_GOES_HERE
  bc3 : xbsIndex_r4/Convert  gui_display_data_type=Fixed-point  arith_type=Boolean  n_bits=1  bin_pt=0  float_type=Single  exp_bits=8  fraction_bits=24  quantization=Truncate  overflow=Wrap  en=off  latency=0  dbl_ovrd=off  pipeline=off  block_type=convert
      settings: xl_area=[0 0 0 0 0 0 0]  block_version=VER_STRING_GOES_HERE
  yn : Outport
  accrm : Outport  Port=2
  wires:
  coeff/1 -> AddSub/2, Gateway Out6/1
  bc2/1 -> AddSub/3
  bc3/1 -> Register2/2
  ld/1 -> bc3/1, Gateway Out2/1
  Register1/1 -> AddSub/1
  Register2/1 -> yn/1, Gateway Out3/1
  AddSub/1 -> Register2/1, Register1/1, accrm/1, Gateway Out8/1
  a/1 -> bc2/1, Gateway Out7/1
  Gateway Out8/1 -> ?
  Gateway Out7/1 -> ?
  Gateway Out6/1 -> ?
  Gateway Out1/1 -> ?
  Gateway Out2/1 -> ?
  Gateway Out3/1 -> ?
  rst/1 -> Register1/2, Gateway Out1/1

== system wlan_phy_rx_pmd/Sync & Antenna Sel/LTS Correlation/Correlator/C4/C6/MAC ==
  a : Inport
  coeff : Inport  Port=2
  ld : Inport  Port=3
  rst : Inport  Port=4
  AddSub : xbsIndex_r4/AddSub  mode=Addition or Subtraction  use_carryin=off  use_carryout=off  en=off  latency=0  precision=User Defined  arith_type="Signed  (2's comp)"  n_bits=long_cor_acc_n_bits  bin_pt=0  quantization=Truncate  overflow=Flag as error  dbl_ovrd=off  use_behavioral_HDL=on  hw_selection=Fabric  pipelined=off  use_rpm=off  block_type=addsub
      settings: xl_area=[2 0 0 4 0 0 0]  block_version=VER_STRING_GOES_HERE
  Gateway Out{1..3,6..8} (6 alike) : xbsIndex_r4/Gateway Out  inherit_from_input=off  hdl_port=off  timing_constraint=None  locs_specified=off  LOCs={}  UseAsDAC=off  DACChannel='1'  block_type=gatewayout
      settings: infoedit="Gateway out block.  Converts Xilinx fixed point inputs into ouputs of type Simulink integer, double, or fixed point.<P><P>Hardware notes:  In hardware these blocks become top level output ports or are discarded, depending on how they are configured."  xl_area=[0 0 0 0 0 0 0]  block_version=VER_STRING_GOES_HERE
  Register1 : xbsIndex_r4/Register  init=0  rst=on  en=off  dbl_ovrd=off  block_type=register
      settings: xl_area=[2 4 0 0 0 0 0]  block_version=VER_STRING_GOES_HERE
  Register2 : xbsIndex_r4/Register  init=0  rst=off  en=on  dbl_ovrd=off  block_type=register
      settings: xl_area=[2 4 0 0 0 0 0]  block_version=VER_STRING_GOES_HERE
  bc2 : xbsIndex_r4/Convert  gui_display_data_type=Fixed-point  arith_type=Boolean  n_bits=1  bin_pt=0  float_type=Single  exp_bits=8  fraction_bits=24  quantization=Truncate  overflow=Wrap  en=off  latency=0  dbl_ovrd=off  pipeline=off  block_type=convert
      settings: xl_area=[0 0 0 0 0 0 0]  block_version=VER_STRING_GOES_HERE
  bc3 : xbsIndex_r4/Convert  gui_display_data_type=Fixed-point  arith_type=Boolean  n_bits=1  bin_pt=0  float_type=Single  exp_bits=8  fraction_bits=24  quantization=Truncate  overflow=Wrap  en=off  latency=0  dbl_ovrd=off  pipeline=off  block_type=convert
      settings: xl_area=[0 0 0 0 0 0 0]  block_version=VER_STRING_GOES_HERE
  yn : Outport
  accrm : Outport  Port=2
  wires:
  rst/1 -> Gateway Out1/1, Register1/2
  Gateway Out3/1 -> ?
  Gateway Out2/1 -> ?
  Gateway Out1/1 -> ?
  Gateway Out6/1 -> ?
  Gateway Out7/1 -> ?
  Gateway Out8/1 -> ?
  a/1 -> Gateway Out7/1, bc2/1
  AddSub/1 -> Gateway Out8/1, accrm/1, Register1/1, Register2/1
  Register2/1 -> Gateway Out3/1, yn/1
  Register1/1 -> AddSub/1
  ld/1 -> Gateway Out2/1, bc3/1
  bc3/1 -> Register2/2
  bc2/1 -> AddSub/3
  coeff/1 -> Gateway Out6/1, AddSub/2

== system wlan_phy_rx_pmd/Sync & Antenna Sel/LTS Correlation/Correlator/C4/C7/MAC ==
  a : Inport
  coeff : Inport  Port=2
  ld : Inport  Port=3
  rst : Inport  Port=4
  AddSub : xbsIndex_r4/AddSub  mode=Addition or Subtraction  use_carryin=off  use_carryout=off  en=off  latency=0  precision=User Defined  arith_type="Signed  (2's comp)"  n_bits=long_cor_acc_n_bits  bin_pt=0  quantization=Truncate  overflow=Flag as error  dbl_ovrd=off  use_behavioral_HDL=on  hw_selection=Fabric  pipelined=off  use_rpm=off  block_type=addsub
      settings: xl_area=[2 0 0 4 0 0 0]  block_version=VER_STRING_GOES_HERE
  Gateway Out{1..3,6..8} (6 alike) : xbsIndex_r4/Gateway Out  inherit_from_input=off  hdl_port=off  timing_constraint=None  locs_specified=off  LOCs={}  UseAsDAC=off  DACChannel='1'  block_type=gatewayout
      settings: infoedit="Gateway out block.  Converts Xilinx fixed point inputs into ouputs of type Simulink integer, double, or fixed point.<P><P>Hardware notes:  In hardware these blocks become top level output ports or are discarded, depending on how they are configured."  xl_area=[0 0 0 0 0 0 0]  block_version=VER_STRING_GOES_HERE
  Register1 : xbsIndex_r4/Register  init=0  rst=on  en=off  dbl_ovrd=off  block_type=register
      settings: xl_area=[2 4 0 0 0 0 0]  block_version=VER_STRING_GOES_HERE
  Register2 : xbsIndex_r4/Register  init=0  rst=off  en=on  dbl_ovrd=off  block_type=register
      settings: xl_area=[2 4 0 0 0 0 0]  block_version=VER_STRING_GOES_HERE
  bc2 : xbsIndex_r4/Convert  gui_display_data_type=Fixed-point  arith_type=Boolean  n_bits=1  bin_pt=0  float_type=Single  exp_bits=8  fraction_bits=24  quantization=Truncate  overflow=Wrap  en=off  latency=0  dbl_ovrd=off  pipeline=off  block_type=convert
      settings: xl_area=[0 0 0 0 0 0 0]  block_version=VER_STRING_GOES_HERE
  bc3 : xbsIndex_r4/Convert  gui_display_data_type=Fixed-point  arith_type=Boolean  n_bits=1  bin_pt=0  float_type=Single  exp_bits=8  fraction_bits=24  quantization=Truncate  overflow=Wrap  en=off  latency=0  dbl_ovrd=off  pipeline=off  block_type=convert
      settings: xl_area=[0 0 0 0 0 0 0]  block_version=VER_STRING_GOES_HERE
  yn : Outport
  accrm : Outport  Port=2
  wires:
  coeff/1 -> AddSub/2, Gateway Out6/1
  bc2/1 -> AddSub/3
  bc3/1 -> Register2/2
  ld/1 -> bc3/1, Gateway Out2/1
  Register1/1 -> AddSub/1
  Register2/1 -> yn/1, Gateway Out3/1
  AddSub/1 -> Register2/1, Register1/1, accrm/1, Gateway Out8/1
  a/1 -> bc2/1, Gateway Out7/1
  Gateway Out8/1 -> ?
  Gateway Out7/1 -> ?
  Gateway Out6/1 -> ?
  Gateway Out1/1 -> ?
  Gateway Out2/1 -> ?
  Gateway Out3/1 -> ?
  rst/1 -> Register1/2, Gateway Out1/1

== system wlan_phy_rx_pmd/Sync & Antenna Sel/LTS Correlation/Correlator/C4/C8/MAC ==
  a : Inport
  coeff : Inport  Port=2
  ld : Inport  Port=3
  rst : Inport  Port=4
  AddSub : xbsIndex_r4/AddSub  mode=Addition or Subtraction  use_carryin=off  use_carryout=off  en=off  latency=0  precision=User Defined  arith_type="Signed  (2's comp)"  n_bits=long_cor_acc_n_bits  bin_pt=0  quantization=Truncate  overflow=Flag as error  dbl_ovrd=off  use_behavioral_HDL=on  hw_selection=Fabric  pipelined=off  use_rpm=off  block_type=addsub
      settings: xl_area=[2 0 0 4 0 0 0]  block_version=VER_STRING_GOES_HERE
  Gateway Out{1..3,6..8} (6 alike) : xbsIndex_r4/Gateway Out  inherit_from_input=off  hdl_port=off  timing_constraint=None  locs_specified=off  LOCs={}  UseAsDAC=off  DACChannel='1'  block_type=gatewayout
      settings: infoedit="Gateway out block.  Converts Xilinx fixed point inputs into ouputs of type Simulink integer, double, or fixed point.<P><P>Hardware notes:  In hardware these blocks become top level output ports or are discarded, depending on how they are configured."  xl_area=[0 0 0 0 0 0 0]  block_version=VER_STRING_GOES_HERE
  Register1 : xbsIndex_r4/Register  init=0  rst=on  en=off  dbl_ovrd=off  block_type=register
      settings: xl_area=[2 4 0 0 0 0 0]  block_version=VER_STRING_GOES_HERE
  Register2 : xbsIndex_r4/Register  init=0  rst=off  en=on  dbl_ovrd=off  block_type=register
      settings: xl_area=[2 4 0 0 0 0 0]  block_version=VER_STRING_GOES_HERE
  bc2 : xbsIndex_r4/Convert  gui_display_data_type=Fixed-point  arith_type=Boolean  n_bits=1  bin_pt=0  float_type=Single  exp_bits=8  fraction_bits=24  quantization=Truncate  overflow=Wrap  en=off  latency=0  dbl_ovrd=off  pipeline=off  block_type=convert
      settings: xl_area=[0 0 0 0 0 0 0]  block_version=VER_STRING_GOES_HERE
  bc3 : xbsIndex_r4/Convert  gui_display_data_type=Fixed-point  arith_type=Boolean  n_bits=1  bin_pt=0  float_type=Single  exp_bits=8  fraction_bits=24  quantization=Truncate  overflow=Wrap  en=off  latency=0  dbl_ovrd=off  pipeline=off  block_type=convert
      settings: xl_area=[0 0 0 0 0 0 0]  block_version=VER_STRING_GOES_HERE
  yn : Outport
  accrm : Outport  Port=2
  wires:
  rst/1 -> Gateway Out1/1, Register1/2
  Gateway Out3/1 -> ?
  Gateway Out2/1 -> ?
  Gateway Out1/1 -> ?
  Gateway Out6/1 -> ?
  Gateway Out7/1 -> ?
  Gateway Out8/1 -> ?
  a/1 -> Gateway Out7/1, bc2/1
  AddSub/1 -> Gateway Out8/1, accrm/1, Register1/1, Register2/1
  Register2/1 -> Gateway Out3/1, yn/1
  Register1/1 -> AddSub/1
  ld/1 -> Gateway Out2/1, bc3/1
  bc3/1 -> Register2/2
  bc2/1 -> AddSub/3
  coeff/1 -> Gateway Out6/1, AddSub/2

== system wlan_phy_rx_pmd/Sync & Antenna Sel/LTS Correlation/Correlator/C5/C1/MAC ==
  a : Inport
  coeff : Inport  Port=2
  ld : Inport  Port=3
  rst : Inport  Port=4
  AddSub : xbsIndex_r4/AddSub  mode=Addition or Subtraction  use_carryin=off  use_carryout=off  en=off  latency=0  precision=User Defined  arith_type="Signed  (2's comp)"  n_bits=long_cor_acc_n_bits  bin_pt=0  quantization=Truncate  overflow=Flag as error  dbl_ovrd=off  use_behavioral_HDL=on  hw_selection=Fabric  pipelined=off  use_rpm=off  block_type=addsub
      settings: xl_area=[2 0 0 4 0 0 0]  block_version=VER_STRING_GOES_HERE
  Gateway Out{1..3,6..8} (6 alike) : xbsIndex_r4/Gateway Out  inherit_from_input=off  hdl_port=off  timing_constraint=None  locs_specified=off  LOCs={}  UseAsDAC=off  DACChannel='1'  block_type=gatewayout
      settings: infoedit="Gateway out block.  Converts Xilinx fixed point inputs into ouputs of type Simulink integer, double, or fixed point.<P><P>Hardware notes:  In hardware these blocks become top level output ports or are discarded, depending on how they are configured."  xl_area=[0 0 0 0 0 0 0]  block_version=VER_STRING_GOES_HERE
  Register1 : xbsIndex_r4/Register  init=0  rst=on  en=off  dbl_ovrd=off  block_type=register
      settings: xl_area=[2 4 0 0 0 0 0]  block_version=VER_STRING_GOES_HERE
  Register2 : xbsIndex_r4/Register  init=0  rst=off  en=on  dbl_ovrd=off  block_type=register
      settings: xl_area=[2 4 0 0 0 0 0]  block_version=VER_STRING_GOES_HERE
  bc2 : xbsIndex_r4/Convert  gui_display_data_type=Fixed-point  arith_type=Boolean  n_bits=1  bin_pt=0  float_type=Single  exp_bits=8  fraction_bits=24  quantization=Truncate  overflow=Wrap  en=off  latency=0  dbl_ovrd=off  pipeline=off  block_type=convert
      settings: xl_area=[0 0 0 0 0 0 0]  block_version=VER_STRING_GOES_HERE
  bc3 : xbsIndex_r4/Convert  gui_display_data_type=Fixed-point  arith_type=Boolean  n_bits=1  bin_pt=0  float_type=Single  exp_bits=8  fraction_bits=24  quantization=Truncate  overflow=Wrap  en=off  latency=0  dbl_ovrd=off  pipeline=off  block_type=convert
      settings: xl_area=[0 0 0 0 0 0 0]  block_version=VER_STRING_GOES_HERE
  yn : Outport
  accrm : Outport  Port=2
  wires:
  rst/1 -> Gateway Out1/1, Register1/2
  Gateway Out3/1 -> ?
  Gateway Out2/1 -> ?
  Gateway Out1/1 -> ?
  Gateway Out6/1 -> ?
  Gateway Out7/1 -> ?
  Gateway Out8/1 -> ?
  a/1 -> Gateway Out7/1, bc2/1
  AddSub/1 -> Gateway Out8/1, accrm/1, Register1/1, Register2/1
  Register2/1 -> Gateway Out3/1, yn/1
  Register1/1 -> AddSub/1
  ld/1 -> Gateway Out2/1, bc3/1
  bc3/1 -> Register2/2
  bc2/1 -> AddSub/3
  coeff/1 -> Gateway Out6/1, AddSub/2

== system wlan_phy_rx_pmd/Sync & Antenna Sel/LTS Correlation/Correlator/C5/C2/MAC ==
  a : Inport
  coeff : Inport  Port=2
  ld : Inport  Port=3
  rst : Inport  Port=4
  AddSub : xbsIndex_r4/AddSub  mode=Addition or Subtraction  use_carryin=off  use_carryout=off  en=off  latency=0  precision=User Defined  arith_type="Signed  (2's comp)"  n_bits=long_cor_acc_n_bits  bin_pt=0  quantization=Truncate  overflow=Flag as error  dbl_ovrd=off  use_behavioral_HDL=on  hw_selection=Fabric  pipelined=off  use_rpm=off  block_type=addsub
      settings: xl_area=[2 0 0 4 0 0 0]  block_version=VER_STRING_GOES_HERE
  Gateway Out{1..3,6..8} (6 alike) : xbsIndex_r4/Gateway Out  inherit_from_input=off  hdl_port=off  timing_constraint=None  locs_specified=off  LOCs={}  UseAsDAC=off  DACChannel='1'  block_type=gatewayout
      settings: infoedit="Gateway out block.  Converts Xilinx fixed point inputs into ouputs of type Simulink integer, double, or fixed point.<P><P>Hardware notes:  In hardware these blocks become top level output ports or are discarded, depending on how they are configured."  xl_area=[0 0 0 0 0 0 0]  block_version=VER_STRING_GOES_HERE
  Register1 : xbsIndex_r4/Register  init=0  rst=on  en=off  dbl_ovrd=off  block_type=register
      settings: xl_area=[2 4 0 0 0 0 0]  block_version=VER_STRING_GOES_HERE
  Register2 : xbsIndex_r4/Register  init=0  rst=off  en=on  dbl_ovrd=off  block_type=register
      settings: xl_area=[2 4 0 0 0 0 0]  block_version=VER_STRING_GOES_HERE
  bc2 : xbsIndex_r4/Convert  gui_display_data_type=Fixed-point  arith_type=Boolean  n_bits=1  bin_pt=0  float_type=Single  exp_bits=8  fraction_bits=24  quantization=Truncate  overflow=Wrap  en=off  latency=0  dbl_ovrd=off  pipeline=off  block_type=convert
      settings: xl_area=[0 0 0 0 0 0 0]  block_version=VER_STRING_GOES_HERE
  bc3 : xbsIndex_r4/Convert  gui_display_data_type=Fixed-point  arith_type=Boolean  n_bits=1  bin_pt=0  float_type=Single  exp_bits=8  fraction_bits=24  quantization=Truncate  overflow=Wrap  en=off  latency=0  dbl_ovrd=off  pipeline=off  block_type=convert
      settings: xl_area=[0 0 0 0 0 0 0]  block_version=VER_STRING_GOES_HERE
  yn : Outport
  accrm : Outport  Port=2
  wires:
  coeff/1 -> AddSub/2, Gateway Out6/1
  bc2/1 -> AddSub/3
  bc3/1 -> Register2/2
  ld/1 -> bc3/1, Gateway Out2/1
  Register1/1 -> AddSub/1
  Register2/1 -> yn/1, Gateway Out3/1
  AddSub/1 -> Register2/1, Register1/1, accrm/1, Gateway Out8/1
  a/1 -> bc2/1, Gateway Out7/1
  Gateway Out8/1 -> ?
  Gateway Out7/1 -> ?
  Gateway Out6/1 -> ?
  Gateway Out1/1 -> ?
  Gateway Out2/1 -> ?
  Gateway Out3/1 -> ?
  rst/1 -> Register1/2, Gateway Out1/1

== system wlan_phy_rx_pmd/Sync & Antenna Sel/LTS Correlation/Correlator/C5/C3/MAC ==
  a : Inport
  coeff : Inport  Port=2
  ld : Inport  Port=3
  rst : Inport  Port=4
  AddSub : xbsIndex_r4/AddSub  mode=Addition or Subtraction  use_carryin=off  use_carryout=off  en=off  latency=0  precision=User Defined  arith_type="Signed  (2's comp)"  n_bits=long_cor_acc_n_bits  bin_pt=0  quantization=Truncate  overflow=Flag as error  dbl_ovrd=off  use_behavioral_HDL=on  hw_selection=Fabric  pipelined=off  use_rpm=off  block_type=addsub
      settings: xl_area=[2 0 0 4 0 0 0]  block_version=VER_STRING_GOES_HERE
  Gateway Out{1..3,6..8} (6 alike) : xbsIndex_r4/Gateway Out  inherit_from_input=off  hdl_port=off  timing_constraint=None  locs_specified=off  LOCs={}  UseAsDAC=off  DACChannel='1'  block_type=gatewayout
      settings: infoedit="Gateway out block.  Converts Xilinx fixed point inputs into ouputs of type Simulink integer, double, or fixed point.<P><P>Hardware notes:  In hardware these blocks become top level output ports or are discarded, depending on how they are configured."  xl_area=[0 0 0 0 0 0 0]  block_version=VER_STRING_GOES_HERE
  Register1 : xbsIndex_r4/Register  init=0  rst=on  en=off  dbl_ovrd=off  block_type=register
      settings: xl_area=[2 4 0 0 0 0 0]  block_version=VER_STRING_GOES_HERE
  Register2 : xbsIndex_r4/Register  init=0  rst=off  en=on  dbl_ovrd=off  block_type=register
      settings: xl_area=[2 4 0 0 0 0 0]  block_version=VER_STRING_GOES_HERE
  bc2 : xbsIndex_r4/Convert  gui_display_data_type=Fixed-point  arith_type=Boolean  n_bits=1  bin_pt=0  float_type=Single  exp_bits=8  fraction_bits=24  quantization=Truncate  overflow=Wrap  en=off  latency=0  dbl_ovrd=off  pipeline=off  block_type=convert
      settings: xl_area=[0 0 0 0 0 0 0]  block_version=VER_STRING_GOES_HERE
  bc3 : xbsIndex_r4/Convert  gui_display_data_type=Fixed-point  arith_type=Boolean  n_bits=1  bin_pt=0  float_type=Single  exp_bits=8  fraction_bits=24  quantization=Truncate  overflow=Wrap  en=off  latency=0  dbl_ovrd=off  pipeline=off  block_type=convert
      settings: xl_area=[0 0 0 0 0 0 0]  block_version=VER_STRING_GOES_HERE
  yn : Outport
  accrm : Outport  Port=2
  wires:
  rst/1 -> Gateway Out1/1, Register1/2
  Gateway Out3/1 -> ?
  Gateway Out2/1 -> ?
  Gateway Out1/1 -> ?
  Gateway Out6/1 -> ?
  Gateway Out7/1 -> ?
  Gateway Out8/1 -> ?
  a/1 -> Gateway Out7/1, bc2/1
  AddSub/1 -> Gateway Out8/1, accrm/1, Register1/1, Register2/1
  Register2/1 -> Gateway Out3/1, yn/1
  Register1/1 -> AddSub/1
  ld/1 -> Gateway Out2/1, bc3/1
  bc3/1 -> Register2/2
  bc2/1 -> AddSub/3
  coeff/1 -> Gateway Out6/1, AddSub/2

== system wlan_phy_rx_pmd/Sync & Antenna Sel/LTS Correlation/Correlator/C5/C4/MAC ==
  a : Inport
  coeff : Inport  Port=2
  ld : Inport  Port=3
  rst : Inport  Port=4
  AddSub : xbsIndex_r4/AddSub  mode=Addition or Subtraction  use_carryin=off  use_carryout=off  en=off  latency=0  precision=User Defined  arith_type="Signed  (2's comp)"  n_bits=long_cor_acc_n_bits  bin_pt=0  quantization=Truncate  overflow=Flag as error  dbl_ovrd=off  use_behavioral_HDL=on  hw_selection=Fabric  pipelined=off  use_rpm=off  block_type=addsub
      settings: xl_area=[2 0 0 4 0 0 0]  block_version=VER_STRING_GOES_HERE
  Gateway Out{1..3,6..8} (6 alike) : xbsIndex_r4/Gateway Out  inherit_from_input=off  hdl_port=off  timing_constraint=None  locs_specified=off  LOCs={}  UseAsDAC=off  DACChannel='1'  block_type=gatewayout
      settings: infoedit="Gateway out block.  Converts Xilinx fixed point inputs into ouputs of type Simulink integer, double, or fixed point.<P><P>Hardware notes:  In hardware these blocks become top level output ports or are discarded, depending on how they are configured."  xl_area=[0 0 0 0 0 0 0]  block_version=VER_STRING_GOES_HERE
  Register1 : xbsIndex_r4/Register  init=0  rst=on  en=off  dbl_ovrd=off  block_type=register
      settings: xl_area=[2 4 0 0 0 0 0]  block_version=VER_STRING_GOES_HERE
  Register2 : xbsIndex_r4/Register  init=0  rst=off  en=on  dbl_ovrd=off  block_type=register
      settings: xl_area=[2 4 0 0 0 0 0]  block_version=VER_STRING_GOES_HERE
  bc2 : xbsIndex_r4/Convert  gui_display_data_type=Fixed-point  arith_type=Boolean  n_bits=1  bin_pt=0  float_type=Single  exp_bits=8  fraction_bits=24  quantization=Truncate  overflow=Wrap  en=off  latency=0  dbl_ovrd=off  pipeline=off  block_type=convert
      settings: xl_area=[0 0 0 0 0 0 0]  block_version=VER_STRING_GOES_HERE
  bc3 : xbsIndex_r4/Convert  gui_display_data_type=Fixed-point  arith_type=Boolean  n_bits=1  bin_pt=0  float_type=Single  exp_bits=8  fraction_bits=24  quantization=Truncate  overflow=Wrap  en=off  latency=0  dbl_ovrd=off  pipeline=off  block_type=convert
      settings: xl_area=[0 0 0 0 0 0 0]  block_version=VER_STRING_GOES_HERE
  yn : Outport
  accrm : Outport  Port=2
  wires:
  coeff/1 -> AddSub/2, Gateway Out6/1
  bc2/1 -> AddSub/3
  bc3/1 -> Register2/2
  ld/1 -> bc3/1, Gateway Out2/1
  Register1/1 -> AddSub/1
  Register2/1 -> yn/1, Gateway Out3/1
  AddSub/1 -> Register2/1, Register1/1, accrm/1, Gateway Out8/1
  a/1 -> bc2/1, Gateway Out7/1
  Gateway Out8/1 -> ?
  Gateway Out7/1 -> ?
  Gateway Out6/1 -> ?
  Gateway Out1/1 -> ?
  Gateway Out2/1 -> ?
  Gateway Out3/1 -> ?
  rst/1 -> Register1/2, Gateway Out1/1
